simulink model "TosAeb"  (R2021a)
settings: Solver=FixedStepDiscrete  StartTime=0.0  StopTime=inf  FixedStep=0.01  RelTol=1e-3
undefined symbols (not defined in the file or in its folder): ACCModSts, AccSts1, AEB_ObjectMovingOrientation, AEB_ObjectMovingState, CN_BTCActive, CN_CurrentGearR, CN_CurrentGearR_DHT, CN_PTCActive, CN_VDCActive, CN_VSC_EspOff, CN_VSC_FCW_AEB_Error, CN_VSC_FCW_AEB_NoDispay, CN_VSC_FCW_AEB_PerfmDgdtn, CN_VSC_FCW_AEB_TempUnAvlbl, CN_VSC_VehStandstill, CNaccelAvoidMax, CNaccelAvoidMin, CNbrakePedalPress, CNcomfortStrLeftAccelMax, CNcomfortStrLeftAccelMin, CNcomfortStrRightAccelMax, CNcomfortStrRightAccelMin, CNDrvDoorOpen, CNDrvModeEco, CNDrvModeNorm, CNDrvModeOther, CNDrvModeSport, CNDrvSBRreminder, CNNull, CNobjInvalidID, CNPassDoorOpen, CNPassSBRreminder, CNPEBSAccelNegInf, CNPEBSAccelNull, CNPEBSTimeInf, CNPEBSTimeNull, CNSTOPMAX, CNSTOPNULL, CNsubjWidth, CNSwtOFF, CNSwtON, CNTTCMAX, CNTTCNULL, CNVehConfigAEBCycNone, CNVehConfigAEBNone, CNVehConfigAEBPedNone, CNVehConfigFCWNone, CNVehConfigJANone, CollisionSeverity, FALSE, FCWSnvty, JACycObjCalcInfoDefaultParams, JAPedObjCalcInfoDefaultParams, JAVehObjCalcInfoDefaultParams, latCycObjCalcInfoDefaultParams, latPedObjCalcInfoDefaultParams, longObjCalcInfoDefaultParams, ModSts, movingStatus, ObjectType ... (+1112)
libraries: simulink x3031, AEBSFunctionLib x50, Ta_Library x33, AEBSStateMachineLib x23, Mylibrary x12, simulink_extras x7, ObjSelect_lib x3, dspstat3 x2
(part 60 of 102)

== system TosAeb/FcwAebJa_Step_sys/ALG_AEBSystem/ALG_AEBController/AEB_FuncCondCalcApp/JaVehFunctionCondCalc/AEBJAVehAEBfullSuppAborCond/AEBJAVehAEBfullSuppCondCalc/AEBJAVehAEBfullSuppSubjCondCalcu/brakeReleventSuppCond/brakeReleventSuppCond_ESP ==
  ESP_MasterCylindBrakePress : Inport
  Action Port : ActionPort  ActionPortLabel=if(u1)
  Compare To Constant12 : simulink/Logic and Bit Operations/Compare To Constant  relop=>  const=P_AEB_Func_JaVehAEBfullMasterCylindBrkPressSupThres  OutDataTypeStr=boolean
  Constant : Constant  Value=P_AEB_Func_JaVehAEBfullBrkCondAborDelayTime
  Constant1 : Constant  Value=Ts
  StateDelayAbortion1 : AEBSFunctionLib/StateDelayAbortion  SystemSampleTime=-1  VariantActivationTime=update diagram  Latency=0  AutoFrameSizeCalculation=off
  brakeReleventSuppCond : Outport
  wires:
  Compare To Constant12/1 -> StateDelayAbortion1/1
  Constant1/1 -> StateDelayAbortion1/3
  Constant/1 -> StateDelayAbortion1/2
  StateDelayAbortion1/1 -> brakeReleventSuppCond/1  "BrkReleventSuppCondFulfilled"
  ESP_MasterCylindBrakePress/1 -> Compare To Constant12/1

== system TosAeb/FcwAebJa_Step_sys/ALG_AEBSystem/ALG_AEBController/AEB_FuncCondCalcApp/JaVehFunctionCondCalc/AEBJAVehAEBfullSuppAborCond/AEBJAVehAEBfullSuppCondCalc/AEBJAVehAEBfullSuppSubjCondCalcu/brakeReleventSuppCond/brakeReleventSuppCond_IBooster ==
  note: 0x0:Not applied
      | 0x1:Applied
  Action Port : ActionPort  ActionPortLabel=else
  Constant : Constant  Value=FALSE
  brakeReleventSuppCond : Outport
  wires:
  Constant/1 -> brakeReleventSuppCond/1  "brakeReleventSuppCond"

== system TosAeb/FcwAebJa_Step_sys/ALG_AEBSystem/ALG_AEBController/AEB_FuncCondCalcApp/JaVehFunctionCondCalc/AEBJAVehPrefillSuppAborCond/AEBJAVehPrefillSuppCondCalc/AEBJAVehPrefillSuppSubjCondCalcu/brakeReleventSuppCond/brakeReleventSuppCond_ESP ==
  BrakePedalStatus_G : Inport
  ESP_MasterCylindBrakePress : Inport  Port=2
  Action Port : ActionPort  ActionPortLabel=if(u1)
  Compare To Constant1 : simulink/Logic and Bit Operations/Compare To Constant  relop===  const=TRUE  OutDataTypeStr=boolean
  Compare To Constant12 : simulink/Logic and Bit Operations/Compare To Constant  relop=>  const=P_AEB_Func_JaVehPrefillMasterCylindBrkPressSupThres  OutDataTypeStr=boolean
  Constant : Constant  Value=P_AEB_Func_JaVehPrefillBrkCondAborDelayTime
  Constant1 : Constant  Value=Ts
  Logical Operator3 : Logic  Operator=OR  AllPortsSameDT=off  OutDataTypeStr=boolean
  StateDelayAbortion1 : AEBSFunctionLib/StateDelayAbortion  SystemSampleTime=-1  VariantActivationTime=update diagram  Latency=0  AutoFrameSizeCalculation=off
  brakeReleventSuppCond : Outport
  wires:
  Logical Operator3/1 -> brakeReleventSuppCond/1
  BrakePedalStatus_G/1 -> Compare To Constant1/1
  Constant1/1 -> StateDelayAbortion1/3
  Constant/1 -> StateDelayAbortion1/2
  StateDelayAbortion1/1 -> Logical Operator3/2
  ESP_MasterCylindBrakePress/1 -> Compare To Constant12/1
  Compare To Constant1/1 -> Logical Operator3/1
  Compare To Constant12/1 -> StateDelayAbortion1/1

== system TosAeb/FcwAebJa_Step_sys/ALG_AEBSystem/ALG_AEBController/AEB_FuncCondCalcApp/JaVehFunctionCondCalc/AEBJAVehPrefillSuppAborCond/AEBJAVehPrefillSuppCondCalc/AEBJAVehPrefillSuppSubjCondCalcu/brakeReleventSuppCond/brakeReleventSuppCond_IBooster ==
  note: 0x0:Not applied
      | 0x1:Applied
  IB_BrkPedalAppld : Inport
  Action Port : ActionPort  ActionPortLabel=else
  Compare To Constant3 : simulink/Logic and Bit Operations/Compare To Constant  relop===  const=TRUE  OutDataTypeStr=boolean
  brakeReleventSuppCond : Outport
  wires:
  IB_BrkPedalAppld/1 -> Compare To Constant3/1
  Compare To Constant3/1 -> brakeReleventSuppCond/1  "brakeReleventSuppCond"

== system TosAeb/FcwAebJa_Step_sys/ALG_AEBSystem/ALG_AEBController/AEB_FuncCondCalcApp/LongFunctionCondCalc/vehAEBfullSuppAborCondCalc/ vehAEBfullSuppCondCalc/vehAEBfullSituationRelActiveCondCalc/overlapThesCalc/If Action Subsystem ==
  dxFrontBumper : Inport
  ObjectFusion : ActionPort  ActionPortLabel=if(u1)
  OverlapThesLookupTable : Lookup_n-D  NumberOfTableDimensions=1  BreakpointsForDimension1=P_AEB_Func_vehAEBfullFusionOverlapActivationThresX  BeginIndexSearchUsingPreviousIndexResult=on  Table=P_AEB_Func_vehAEBfullFusionOverlapActivationThresY  ExtrapMethod=Clip  InternalRulePriority=Speed  RndMeth=Simplest  InputSameDT=on
  overlapThes : Outport
  wires:
  OverlapThesLookupTable/1 -> overlapThes/1
  dxFrontBumper/1 -> OverlapThesLookupTable/1

== system TosAeb/FcwAebJa_Step_sys/ALG_AEBSystem/ALG_AEBController/AEB_FuncCondCalcApp/LongFunctionCondCalc/vehAEBfullSuppAborCondCalc/ vehAEBfullSuppCondCalc/vehAEBfullSituationRelActiveCondCalc/overlapThesCalc/If Action Subsystem1 ==
  dxFrontBumper : Inport
  ObjectNoFusion : ActionPort  ActionPortLabel=else
  OverlapThesLookupTable : Lookup_n-D  NumberOfTableDimensions=1  BreakpointsForDimension1=P_AEB_Func_vehAEBfullNoFusionOverlapActivationThresX  BeginIndexSearchUsingPreviousIndexResult=on  Table=P_AEB_Func_vehAEBfullNoFusionOverlapActivationThresThresY  ExtrapMethod=Clip  InternalRulePriority=Speed  RndMeth=Simplest  InputSameDT=on
  overlapThes : Outport
  wires:
  dxFrontBumper/1 -> OverlapThesLookupTable/1
  OverlapThesLookupTable/1 -> overlapThes/1

== system TosAeb/FcwAebJa_Step_sys/ALG_AEBSystem/ALG_AEBController/AEB_FuncCondCalcApp/LongFunctionCondCalc/vehAEBfullSuppAborCondCalc/ vehAEBfullSuppCondCalc/vehAEBfullVehRelActiveCondCalc/DriverCondJudge/brakeReleventSuppCond ==
  VehConfigIbooster : Inport
  ESPmasterCylindBrakePress : Inport  Port=2
  BrakePedalStatus_G : Inport  Port=3
  subjLongAccel : Inport  Port=4
  subjVel : Inport  Port=5
  Compare To Constant : simulink/Logic and Bit Operations/Compare To Constant  relop===  const=FALSE  OutDataTypeStr=boolean
  If : If  IfExpression=u1
  Merge : Merge
  brakeReleventSuppCond_ESP : Subsystem  VariantControl=(default)
  brakeReleventSuppCond_IBooster : Subsystem  VariantControl=P_AEB_PowerTrainConfig == 1
  brakeReleventSuppCond : Outport
  wires:
  subjVel/1 -> brakeReleventSuppCond_ESP/4(subjVel)
  subjLongAccel/1 -> brakeReleventSuppCond_ESP/3(subjLongAccel)
  BrakePedalStatus_G/1 -> brakeReleventSuppCond_ESP/2(BrakePedalStatus_G)
  ESPmasterCylindBrakePress/1 -> brakeReleventSuppCond_ESP/1(ESPmasterCylindBrakePress)
  Merge/1 -> brakeReleventSuppCond/1
  brakeReleventSuppCond_IBooster/1(brakeReleventSuppCond) -> Merge/2
  brakeReleventSuppCond_ESP/1(brakeReleventSuppCond) -> Merge/1
  If/2 -> brakeReleventSuppCond_IBooster/Action
  If/1 -> brakeReleventSuppCond_ESP/Action
  Compare To Constant/1 -> If/1
  VehConfigIbooster/1 -> Compare To Constant/1

== system TosAeb/FcwAebJa_Step_sys/ALG_AEBSystem/ALG_AEBController/AEB_FuncCondCalcApp/LongFunctionCondCalc/vehAEBpartSuppAborCondCalc/vehAEBpartSuppCondCalc/vehAEBpartSituationRelActiveCondCalc/overlapThesCalc/If Action Subsystem ==
  dxFrontBumper : Inport
  ObjectFusion : ActionPort  ActionPortLabel=if(u1)
  OverlapThesLookupTable : Lookup_n-D  NumberOfTableDimensions=1  BreakpointsForDimension1=P_AEB_Func_vehAEBpartFusionOverlapActivationThresX  BeginIndexSearchUsingPreviousIndexResult=on  Table=P_AEB_Func_vehAEBpartFusionOverlapActivationThresY  ExtrapMethod=Clip  InternalRulePriority=Speed  RndMeth=Simplest  InputSameDT=on
  overlapThes : Outport
  wires:
  OverlapThesLookupTable/1 -> overlapThes/1
  dxFrontBumper/1 -> OverlapThesLookupTable/1

== system TosAeb/FcwAebJa_Step_sys/ALG_AEBSystem/ALG_AEBController/AEB_FuncCondCalcApp/LongFunctionCondCalc/vehAEBpartSuppAborCondCalc/vehAEBpartSuppCondCalc/vehAEBpartSituationRelActiveCondCalc/overlapThesCalc/If Action Subsystem1 ==
  dxFrontBumper : Inport
  ObjectNoFusion : ActionPort  ActionPortLabel=else
  OverlapThesLookupTable : Lookup_n-D  NumberOfTableDimensions=1  BreakpointsForDimension1=P_AEB_Func_vehAEBpartNoFusionOverlapActivationThresX  BeginIndexSearchUsingPreviousIndexResult=on  Table=P_AEB_Func_vehAEBpartNoFusionOverlapActivationThresY  ExtrapMethod=Clip  InternalRulePriority=Speed  RndMeth=Simplest  InputSameDT=on
  overlapThes : Outport
  wires:
  dxFrontBumper/1 -> OverlapThesLookupTable/1
  OverlapThesLookupTable/1 -> overlapThes/1

== system TosAeb/FcwAebJa_Step_sys/ALG_AEBSystem/ALG_AEBController/AEB_FuncCondCalcApp/LongFunctionCondCalc/vehAEBpartSuppAborCondCalc/vehAEBpartSuppCondCalc/vehAEBpartVehRelActiveCondCalc/DriverCondJudge/brakeReleventSuppCond ==
  VehConfigIbooster : Inport
  ESPmasterCylindBrakePress : Inport  Port=2
  BrakePedalStatus_G : Inport  Port=3
  IB_BrkPedalAppld : Inport  Port=4
  Compare To Constant : simulink/Logic and Bit Operations/Compare To Constant  relop===  const=FALSE  OutDataTypeStr=boolean
  If : If  IfExpression=u1
  Merge : Merge
  brakeReleventSuppCond_ESP : Subsystem  VariantControl=(default)
  brakeReleventSuppCond_IBooster : Subsystem  VariantControl=P_AEB_PowerTrainConfig == 1
  brakeReleventSuppCond : Outport
  wires:
  IB_BrkPedalAppld/1 -> brakeReleventSuppCond_IBooster/1(IB_BrkPedalAppld)
  ESPmasterCylindBrakePress/1 -> brakeReleventSuppCond_ESP/1(ESPmasterCylindBrakePress)
  BrakePedalStatus_G/1 -> brakeReleventSuppCond_ESP/2(BrakePedalStatus_G)
  Merge/1 -> brakeReleventSuppCond/1
  brakeReleventSuppCond_IBooster/1(brakeReleventSuppCond) -> Merge/2
  brakeReleventSuppCond_ESP/1(brakeReleventSuppCond) -> Merge/1
  If/2 -> brakeReleventSuppCond_IBooster/Action
  If/1 -> brakeReleventSuppCond_ESP/Action
  Compare To Constant/1 -> If/1
  VehConfigIbooster/1 -> Compare To Constant/1

== system TosAeb/FcwAebJa_Step_sys/ALG_AEBSystem/ALG_AEBController/AEB_FuncCondCalcApp/LongFunctionCondCalc/vehAcuteWarningSuppAborCondFulfilled/vehAcuteWarningSuppCondCalc/vehAcuteWarningVehRelActiveCondCalc/DriverCondJudge/brakeReleventSuppCond ==
  VehConfigIbooster : Inport
  ESPmasterCylindBrakePress : Inport  Port=2
  BrakePedalStatus_G : Inport  Port=3
  IB_BrkPedalAppld : Inport  Port=4
  Compare To Constant : simulink/Logic and Bit Operations/Compare To Constant  relop===  const=FALSE  OutDataTypeStr=boolean
  If : If  IfExpression=u1
  Merge : Merge
  brakeReleventSuppCond_ESP : Subsystem  VariantControl=(default)
  brakeReleventSuppCond_IBooster : Subsystem  VariantControl=P_AEB_PowerTrainConfig == 1
  brakeReleventSuppCond : Outport
  wires:
  IB_BrkPedalAppld/1 -> brakeReleventSuppCond_IBooster/1(IB_BrkPedalAppld)
  ESPmasterCylindBrakePress/1 -> brakeReleventSuppCond_ESP/1(ESPmasterCylindBrakePress)
  BrakePedalStatus_G/1 -> brakeReleventSuppCond_ESP/2(BrakePedalStatus_G)
  Merge/1 -> brakeReleventSuppCond/1
  brakeReleventSuppCond_IBooster/1(brakeReleventSuppCond) -> Merge/2
  brakeReleventSuppCond_ESP/1(brakeReleventSuppCond) -> Merge/1
  If/2 -> brakeReleventSuppCond_IBooster/Action
  If/1 -> brakeReleventSuppCond_ESP/Action
  Compare To Constant/1 -> If/1
  VehConfigIbooster/1 -> Compare To Constant/1

== system TosAeb/FcwAebJa_Step_sys/ALG_AEBSystem/ALG_AEBController/AEB_FuncCondCalcApp/LongFunctionCondCalc/vehEBASuppAborCondCalc/vehEBASuppCondCalc/vehEBASituationRelActiveCondCalc/brakeReleventSuppCond/brakeReleventSuppCond_ESP ==
  predictiveAccelAvoid : Inport
  ObjectMovingState : Inport  Port=2
  subjVel : Inport  Port=3
  ESPmasterCylindBrakePress : Inport  Port=4
  BrakePedalStatus_G : Inport  Port=5
  subjLongAccelFromSensor : Inport  Port=6
  subjLongAccel : Inport  Port=7
  Action Port : ActionPort  ActionPortLabel=if(u1)
  Constant2 : Constant  Value=P_AEB_Func_vehEBAMasterCylindBrkPressActivationThres
  Constant4 : Constant  Value=P_AEB_Func_vehEBAObjStandAccelAvoidThres
  Constant6 : Constant  Value=P_AEB_Func_vehEBAObjStandBrkAccelThresHigh
  Constant8 : Constant  Value=P_AEB_Func_vehEBAObjStandBrkAccelThresLow
  Constant9 : Constant  Value=P_AEB_Func_vehEBASubjLongAccelActivationThres
  brkTriggerCondCalc1 : Stateflow chart  TreatAsAtomicUnit=on
  brkTriggerCondFulfilled : Outport
  wires:
  ESPmasterCylindBrakePress/1 -> brkTriggerCondCalc1/4(ESPmasterCylindBrakePress)
  brkTriggerCondCalc1/1(brkTriggerCondFulfilled) -> brkTriggerCondFulfilled/1  "brkTriggerCondFulfilled"
  Constant8/1 -> brkTriggerCondCalc1/11(objStandBrkAccelThesLow)
  Constant2/1 -> brkTriggerCondCalc1/8(masterCylindBrkPressThes)
  ObjectMovingState/1 -> brkTriggerCondCalc1/2(objMovingState)
  Constant6/1 -> brkTriggerCondCalc1/10(objStandBrkAccelThesHigh)
  BrakePedalStatus_G/1 -> brkTriggerCondCalc1/5(brakePedalStatus)
  Constant4/1 -> brkTriggerCondCalc1/9(objStandAccelAvoidPredictThes)
  subjLongAccelFromSensor/1 -> brkTriggerCondCalc1/6(subjLongAccelFromSensor)
  Constant9/1 -> brkTriggerCondCalc1/12(subjLongAccelThes)
  subjLongAccel/1 -> brkTriggerCondCalc1/7(subjLongAccel)
  predictiveAccelAvoid/1 -> brkTriggerCondCalc1/1(predictiveAccelAvoid)
  subjVel/1 -> brkTriggerCondCalc1/3(subjVel)

== system TosAeb/FcwAebJa_Step_sys/ALG_AEBSystem/ALG_AEBController/AEB_FuncCondCalcApp/LongFunctionCondCalc/vehEBASuppAborCondCalc/vehEBASuppCondCalc/vehEBASituationRelActiveCondCalc/brakeReleventSuppCond/brakeReleventSuppCond_IBooster ==
  note: 0x0:Not applied
      | 0x1:Applied
  predictiveAccelAvoid : Inport
  ObjectMovingState : Inport  Port=2
  subjVel : Inport  Port=3
  subjLongAccelFromSensor : Inport  Port=4
  subjLongAccel : Inport  Port=5
  IB_BrkPedalAppld : Inport  Port=6
  Action Port : ActionPort  ActionPortLabel=else
  Constant4 : Constant  Value=P_AEB_Func_vehEBAObjStandAccelAvoidThres
  Constant6 : Constant  Value=P_AEB_Func_vehEBAObjStandBrkAccelThresHigh
  Constant8 : Constant  Value=P_AEB_Func_vehEBAObjStandBrkAccelThresLow
  Constant9 : Constant  Value=P_AEB_Func_vehEBASubjLongAccelActivationThres
  IB_BrkPedalAppldOpt : Subsystem
  brkAccelThesLookup : Subsystem  Commented=on
      settings: UndoDeleteFcn=Stateflow.SLINSF.SimulinkMan.restorePreDeleteFcn  PreDeleteFcn=Stateflow.SLINSF.SimulinkMan.removePreDeleteFcn
  brkTriggerCondCalc1 : Stateflow chart  TreatAsAtomicUnit=on
  brkTriggerCondFulfilled : Outport
  wires:
  subjLongAccel/1 -> brkTriggerCondCalc1/5(subjLongAccel)
  Constant4/1 -> brkTriggerCondCalc1/7(objStandAccelAvoidPredictThes)
  subjLongAccelFromSensor/1 -> brkTriggerCondCalc1/4(subjLongAccelFromSensor)
  brkTriggerCondCalc1/1(brkTriggerCondFulfilled) -> brkTriggerCondFulfilled/1  "brkTriggerCondFulfilled"
  Constant9/1 -> brkTriggerCondCalc1/10(subjLongAccelThes)
  Constant6/1 -> brkTriggerCondCalc1/8(objStandBrkAccelThesHigh)
  predictiveAccelAvoid/1 -> brkTriggerCondCalc1/1(predictiveAccelAvoid)
  Constant8/1 -> brkTriggerCondCalc1/9(objStandBrkAccelThesLow)
  ObjectMovingState/1 -> brkTriggerCondCalc1/2(objMovingState)
  subjVel/1 -> brkTriggerCondCalc1/3(subjVel)
  IB_BrkPedalAppld/1 -> IB_BrkPedalAppldOpt/1(IB_BrkPedalAppld)
  IB_BrkPedalAppldOpt/1(IB_BrkPedalAppldOpt) -> brkTriggerCondCalc1/6(IB_BrkPedalAppld)  "AEB_EbaIB_BrkPedalAppldOpt"

== system TosAeb/FcwAebJa_Step_sys/ALG_AEBSystem/ALG_AEBController/AEB_FuncCondCalcApp/LongFunctionCondCalc/vehEBASuppAborCondCalc/vehEBASuppCondCalc/vehEBASituationRelActiveCondCalc/overlapThesCalc/If Action Subsystem ==
  dxFrontBumper : Inport
  ObjectFusion : ActionPort  ActionPortLabel=if(u1)
  OverlapThesLookupTable : Lookup_n-D  NumberOfTableDimensions=1  BreakpointsForDimension1=P_AEB_Func_vehEBAFusionOverlapActivationThresX  BeginIndexSearchUsingPreviousIndexResult=on  Table=P_AEB_Func_vehEBAFusionOverlapActivationThresY  ExtrapMethod=Clip  InternalRulePriority=Speed  RndMeth=Simplest  InputSameDT=on
  overlapThes : Outport
  wires:
  OverlapThesLookupTable/1 -> overlapThes/1
  dxFrontBumper/1 -> OverlapThesLookupTable/1

== system TosAeb/FcwAebJa_Step_sys/ALG_AEBSystem/ALG_AEBController/AEB_FuncCondCalcApp/LongFunctionCondCalc/vehEBASuppAborCondCalc/vehEBASuppCondCalc/vehEBASituationRelActiveCondCalc/overlapThesCalc/If Action Subsystem1 ==
  dxFrontBumper : Inport
  ObjectNoFusion : ActionPort  ActionPortLabel=else
  OverlapThesLookupTable : Lookup_n-D  NumberOfTableDimensions=1  BreakpointsForDimension1=P_AEB_Func_vehEBANoFusionOverlapActivationThresX  BeginIndexSearchUsingPreviousIndexResult=on  Table=P_AEB_Func_vehEBANoFusionOverlapActivationThresY  ExtrapMethod=Clip  InternalRulePriority=Speed  RndMeth=Simplest  InputSameDT=on
  overlapThes : Outport
  wires:
  dxFrontBumper/1 -> OverlapThesLookupTable/1
  OverlapThesLookupTable/1 -> overlapThes/1

== system TosAeb/FcwAebJa_Step_sys/ALG_AEBSystem/ALG_AEBController/AEB_FuncCondCalcApp/LongFunctionCondCalc/vehLatentWarningSuppAborCondFulfilled/vehLatentWarningAborCondCalc/vehLatentWarningSituationRelAborCondCalc/timer/If Action Subsystem2 ==
  Action Port : ActionPort  InitializeStates=reset  ActionPortLabel=if(u1)
  Add : Sum
  Constant : Constant  Value=0.02
  Unit Delay : UnitDelay  InitialCondition=0.02  SampleTime=-1  HasFrameUpgradeWarning=on
  time : Outport
  wires:
  Constant/1 -> Add/2
  Add/1 -> Unit Delay/1
  Unit Delay/1 -> Add/1, time/1

== system TosAeb/FcwAebJa_Step_sys/ALG_AEBSystem/ALG_AEBController/AEB_FuncCondCalcApp/LongFunctionCondCalc/vehLatentWarningSuppAborCondFulfilled/vehLatentWarningAborCondCalc/vehLatentWarningSituationRelAborCondCalc/timer/If Action Subsystem3 ==
  Action Port : ActionPort  ActionPortLabel=else
  Constant : Constant  Value=0
  time : Outport
  wires:
  Constant/1 -> time/1

== system TosAeb/FcwAebJa_Step_sys/ALG_AEBSystem/ALG_AEBController/AEB_FuncCondCalcApp/LongFunctionCondCalc/vehPreWarningSuppAborCondFulfilled/vehPreWarningSuppCondCalc/vehPreWarningSituationRelActiveCondCalc/IsTtcCondFulfilledCalc/LowLvlBrkAccelThesCalcHMIEarly ==
  subjVel : Inport
  Action Port : ActionPort  ActionPortLabel=case [ HMISettingEarly ]:
  AEB_Func_VehPreWarning_TTC_Thres : Lookup_n-D  NumberOfTableDimensions=1  BreakpointsForDimension1=P_AEB_Func_vehPreWarning_TTC_ThresX4Eary  Table=P_AEB_Func_vehPreWarning_TTC_ThresY4Eary  ExtrapMethod=Clip  InternalRulePriority=Speed  RndMeth=Simplest  InputSameDT=on
  VehPreWarning_TTC_Thres : Outport
  wires:
  AEB_Func_VehPreWarning_TTC_Thres/1 -> VehPreWarning_TTC_Thres/1
  subjVel/1 -> AEB_Func_VehPreWarning_TTC_Thres/1

== system TosAeb/FcwAebJa_Step_sys/ALG_AEBSystem/ALG_AEBController/AEB_FuncCondCalcApp/LongFunctionCondCalc/vehPreWarningSuppAborCondFulfilled/vehPreWarningSuppCondCalc/vehPreWarningSituationRelActiveCondCalc/IsTtcCondFulfilledCalc/LowLvlBrkAccelThesCalcHMILate ==
  subjVel : Inport
  Action Port : ActionPort  ActionPortLabel=case [ HMISettingLate ]:
  AEB_Func_VehPreWarning_TTC_Thres : Lookup_n-D  NumberOfTableDimensions=1  BreakpointsForDimension1=P_AEB_Func_vehPreWarning_TTC_ThresX4Late  Table=P_AEB_Func_vehPreWarning_TTC_ThresY4Late  ExtrapMethod=Clip  InternalRulePriority=Speed  RndMeth=Simplest  InputSameDT=on
  VehPreWarning_TTC_Thres : Outport
  wires:
  AEB_Func_VehPreWarning_TTC_Thres/1 -> VehPreWarning_TTC_Thres/1
  subjVel/1 -> AEB_Func_VehPreWarning_TTC_Thres/1

== system TosAeb/FcwAebJa_Step_sys/ALG_AEBSystem/ALG_AEBController/AEB_FuncCondCalcApp/LongFunctionCondCalc/vehPreWarningSuppAborCondFulfilled/vehPreWarningSuppCondCalc/vehPreWarningSituationRelActiveCondCalc/IsTtcCondFulfilledCalc/LowLvlBrkAccelThesCalcHMIMid ==
  subjVel : Inport
  Action Port : ActionPort  ActionPortLabel=default:
  AEB_Func_VehPreWarning_TTC_Thres : Lookup_n-D  NumberOfTableDimensions=1  BreakpointsForDimension1=P_AEB_Func_vehPreWarning_TTC_ThresX4Mid  Table=P_AEB_Func_vehPreWarning_TTC_ThresY4Mid  ExtrapMethod=Clip  InternalRulePriority=Speed  RndMeth=Simplest  InputSameDT=on
  VehPreWarning_TTC_Thres : Outport
  wires:
  AEB_Func_VehPreWarning_TTC_Thres/1 -> VehPreWarning_TTC_Thres/1
  subjVel/1 -> AEB_Func_VehPreWarning_TTC_Thres/1

== system TosAeb/FcwAebJa_Step_sys/ALG_AEBSystem/ALG_AEBController/AEB_FuncCondCalcApp/LongFunctionCondCalc/vehPreWarningSuppAborCondFulfilled/vehPreWarningSuppCondCalc/vehPreWarningVehRelActiveCondCalc/DriverCondJudge/brakeReleventSuppCond ==
  VehConfigIbooster : Inport
  ESPmasterCylindBrakePress : Inport  Port=2
  BrakePedalStatus_G : Inport  Port=3
  IB_BrkPedalAppld : Inport  Port=4
  Compare To Constant : simulink/Logic and Bit Operations/Compare To Constant  relop===  const=FALSE  OutDataTypeStr=boolean
  If : If  IfExpression=u1
  Merge : Merge
  brakeReleventSuppCond_ESP : Subsystem  VariantControl=(default)
  brakeReleventSuppCond_IBooster : Subsystem  VariantControl=P_AEB_PowerTrainConfig == 1
  brakeReleventSuppCond : Outport
  wires:
  IB_BrkPedalAppld/1 -> brakeReleventSuppCond_IBooster/1(IB_BrkPedalAppld)
  ESPmasterCylindBrakePress/1 -> brakeReleventSuppCond_ESP/1(ESPmasterCylindBrakePress)
  BrakePedalStatus_G/1 -> brakeReleventSuppCond_ESP/2(BrakePedalStatus_G)
  Merge/1 -> brakeReleventSuppCond/1
  brakeReleventSuppCond_IBooster/1(brakeReleventSuppCond) -> Merge/2
  brakeReleventSuppCond_ESP/1(brakeReleventSuppCond) -> Merge/1
  If/2 -> brakeReleventSuppCond_IBooster/Action
  If/1 -> brakeReleventSuppCond_ESP/Action
  Compare To Constant/1 -> If/1
  VehConfigIbooster/1 -> Compare To Constant/1

== system TosAeb/FcwAebJa_Step_sys/ALG_AEBSystem/ALG_AEBController/AEB_FuncCondCalcApp/LongFunctionCondCalc/vehPrefillSuppAborCondCalc/vehPrefillSuppCondCalc/vehPrefillVehRelActiveCondCalc/DriverCondJudge/brakeReleventSuppCond ==
  VehConfigIbooster : Inport
  ESPmasterCylindBrakePress : Inport  Port=2
  BrakePedalStatus_G : Inport  Port=3
  IB_BrkPedalAppld : Inport  Port=4
  Compare To Constant : simulink/Logic and Bit Operations/Compare To Constant  relop===  const=FALSE  OutDataTypeStr=boolean
  If : If  IfExpression=u1
  Merge : Merge
  brakeReleventSuppCond_ESP : Subsystem  VariantControl=(default)
  brakeReleventSuppCond_IBooster : Subsystem  VariantControl=P_AEB_PowerTrainConfig == 1
  brakeReleventSuppCond : Outport
  wires:
  IB_BrkPedalAppld/1 -> brakeReleventSuppCond_IBooster/1(IB_BrkPedalAppld)
  ESPmasterCylindBrakePress/1 -> brakeReleventSuppCond_ESP/1(ESPmasterCylindBrakePress)
  BrakePedalStatus_G/1 -> brakeReleventSuppCond_ESP/2(BrakePedalStatus_G)
  Merge/1 -> brakeReleventSuppCond/1
  brakeReleventSuppCond_IBooster/1(brakeReleventSuppCond) -> Merge/2
  brakeReleventSuppCond_ESP/1(brakeReleventSuppCond) -> Merge/1
  If/2 -> brakeReleventSuppCond_IBooster/Action
  If/1 -> brakeReleventSuppCond_ESP/Action
  Compare To Constant/1 -> If/1
  VehConfigIbooster/1 -> Compare To Constant/1

== system TosAeb/FcwAebJa_Step_sys/ALG_AEBSystem/ALG_AEBController/AEB_FuncCondCalcApp/PedFunctionCondCalc/pedAEBfullSuppAborCond/pedAEBfullSuppCondCalc/pedAEBfullSituationRelSuppCondCalc/latDistCondCalc/LateralDistanceThresHMISettingEarly ==
  subjVel : Inport
  HMISettingEarlyOrMiddle : ActionPort  ActionPortLabel=case [ HMISettingEarly HMISettingMiddle ]:
  LateralDistanceThresLookupTable : Lookup_n-D  NumberOfTableDimensions=1  BreakpointsForDimension1=P_AEB_Func_pedAEBfullSubjVelLatBoundHmiEarlyMidX  BeginIndexSearchUsingPreviousIndexResult=on  Table=P_AEB_Func_pedAEBfullDyLatBoundHmiEarlyMidY  ExtrapMethod=Clip  InternalRulePriority=Speed  RndMeth=Simplest  InputSameDT=on
  latDistThres : Outport
  wires:
  subjVel/1 -> LateralDistanceThresLookupTable/1
  LateralDistanceThresLookupTable/1 -> latDistThres/1

== system TosAeb/FcwAebJa_Step_sys/ALG_AEBSystem/ALG_AEBController/AEB_FuncCondCalcApp/PedFunctionCondCalc/pedAEBfullSuppAborCond/pedAEBfullSuppCondCalc/pedAEBfullSituationRelSuppCondCalc/latDistCondCalc/LateralDistanceThresHMISettingLate ==
  vy : Inport
  HMISettingLate : ActionPort  ActionPortLabel=default:
  Abs : Abs
  LateralDistanceThresLookupTable : Lookup_n-D  NumberOfTableDimensions=1  BreakpointsForDimension1=P_AEB_Func_pedAEBfullObjVyAbsLatBoundHmiLateX  BeginIndexSearchUsingPreviousIndexResult=on  Table=P_AEB_Func_pedAEBfullDyLatBoundHmiLateY  ExtrapMethod=Clip  InternalRulePriority=Speed  RndMeth=Simplest  InputSameDT=on
  latDistThres : Outport
  wires:
  Abs/1 -> LateralDistanceThresLookupTable/1
  vy/1 -> Abs/1
  LateralDistanceThresLookupTable/1 -> latDistThres/1

== system TosAeb/FcwAebJa_Step_sys/ALG_AEBSystem/ALG_AEBController/AEB_FuncCondCalcApp/PedFunctionCondCalc/pedAEBfullSuppAborCond/pedAEBfullSuppCondCalc/pedAEBfullVehRelSuppCondCalc/brakeReleventSuppCond/brakeReleventSuppCond_ESP ==
  ESP_MasterCylindBrakePressValid : Inport
  ESP_MasterCylindBrakePress : Inport  Port=2
  Action Port : ActionPort  ActionPortLabel=if(u1)
  Compare To Constant11 : simulink/Logic and Bit Operations/Compare To Constant  relop===  const=TRUE  OutDataTypeStr=boolean
  Compare To Constant12 : simulink/Logic and Bit Operations/Compare To Constant  relop=>  const=P_AEB_Func_pedAEBfullMasterCylindBrkPressSupThres  OutDataTypeStr=boolean
  Logical Operator1 : Logic  AllPortsSameDT=off  OutDataTypeStr=boolean
  brakeReleventSuppCond : Outport
  wires:
  ESP_MasterCylindBrakePress/1 -> Compare To Constant12/1
  ESP_MasterCylindBrakePressValid/1 -> Compare To Constant11/1
  Compare To Constant11/1 -> Logical Operator1/1
  Logical Operator1/1 -> brakeReleventSuppCond/1
  Compare To Constant12/1 -> Logical Operator1/2

== system TosAeb/FcwAebJa_Step_sys/ALG_AEBSystem/ALG_AEBController/AEB_FuncCondCalcApp/PedFunctionCondCalc/pedAEBfullSuppAborCond/pedAEBfullSuppCondCalc/pedAEBfullVehRelSuppCondCalc/brakeReleventSuppCond/brakeReleventSuppCond_IBooster ==
  note: 0x0:Not applied
      | 0x1:Applied
  Action Port : ActionPort  ActionPortLabel=else
  Constant : Constant  Value=FALSE
  brakeReleventSuppCond : Outport
  wires:
  Constant/1 -> brakeReleventSuppCond/1  "brakeReleventSuppCond"

== system TosAeb/FcwAebJa_Step_sys/ALG_AEBSystem/ALG_AEBController/AEB_FuncCondCalcApp/PedFunctionCondCalc/pedPreWarningSuppAborCond/pedPreWarningSuppCondCalc/pedPreWarningVehRelActiveCondCalc/brakeReleventSuppCond/brakeReleventSuppCond_ESP ==
  ESP_MasterCylindBrakePressValid : Inport
  ESP_MasterCylindBrakePress : Inport  Port=2
  Action Port : ActionPort  ActionPortLabel=if(u1)
  Compare To Constant11 : simulink/Logic and Bit Operations/Compare To Constant  relop===  const=TRUE  OutDataTypeStr=boolean
  Compare To Constant12 : simulink/Logic and Bit Operations/Compare To Constant  relop=>  const=P_AEB_Func_pedPrewarningMasterCylindBrkPressSupThres  OutDataTypeStr=boolean
  Logical Operator1 : Logic  AllPortsSameDT=off  OutDataTypeStr=boolean
  brakeReleventSuppCond : Outport
  wires:
  Compare To Constant12/1 -> Logical Operator1/2
  ESP_MasterCylindBrakePressValid/1 -> Compare To Constant11/1
  Compare To Constant11/1 -> Logical Operator1/1
  Logical Operator1/1 -> brakeReleventSuppCond/1
  ESP_MasterCylindBrakePress/1 -> Compare To Constant12/1

== system TosAeb/FcwAebJa_Step_sys/ALG_AEBSystem/ALG_AEBController/AEB_FuncCondCalcApp/PedFunctionCondCalc/pedPreWarningSuppAborCond/pedPreWarningSuppCondCalc/pedPreWarningVehRelActiveCondCalc/brakeReleventSuppCond/brakeReleventSuppCond_IBooster ==
  note: 0x0:Not applied
      | 0x1:Applied
  IB_BrkPedalAppld : Inport
  Action Port : ActionPort  ActionPortLabel=else
  Compare To Constant3 : simulink/Logic and Bit Operations/Compare To Constant  relop===  const=TRUE  OutDataTypeStr=boolean
  brakeReleventSuppCond : Outport
  wires:
  IB_BrkPedalAppld/1 -> Compare To Constant3/1
  Compare To Constant3/1 -> brakeReleventSuppCond/1  "brakeReleventSuppCond"

== system TosAeb/FcwAebJa_Step_sys/ALG_AEBSystem/ALG_AEBController/AEB_FuncCondCalcApp/PedFunctionCondCalc/pedPrefillSuppAborCond/pedPrefillSuppCondCalc/pedPrefillVehRelActiveCondCalc/brakeReleventSuppCond/brakeReleventSuppCond_ESP ==
  ESP_MasterCylindBrakePressValid : Inport
  ESP_MasterCylindBrakePress : Inport  Port=2
  Action Port : ActionPort  ActionPortLabel=if(u1)
  Compare To Constant11 : simulink/Logic and Bit Operations/Compare To Constant  relop===  const=TRUE  OutDataTypeStr=boolean
  Compare To Constant12 : simulink/Logic and Bit Operations/Compare To Constant  relop=>  const=P_AEB_Func_pedPrefillMasterCylindBrkPressSupThres  OutDataTypeStr=boolean
  Logical Operator1 : Logic  AllPortsSameDT=off  OutDataTypeStr=boolean
  brakeReleventSuppCond : Outport
  wires:
  Compare To Constant11/1 -> Logical Operator1/1
  Logical Operator1/1 -> brakeReleventSuppCond/1
  ESP_MasterCylindBrakePress/1 -> Compare To Constant12/1
  ESP_MasterCylindBrakePressValid/1 -> Compare To Constant11/1
  Compare To Constant12/1 -> Logical Operator1/2

== system TosAeb/FcwAebJa_Step_sys/ALG_AEBSystem/ALG_AEBController/AEB_FuncCondCalcApp/PedFunctionCondCalc/pedPrefillSuppAborCond/pedPrefillSuppCondCalc/pedPrefillVehRelActiveCondCalc/brakeReleventSuppCond/brakeReleventSuppCond_IBooster ==
  note: 0x0:Not applied
      | 0x1:Applied
  IB_BrkPedalAppld : Inport
  Action Port : ActionPort  ActionPortLabel=else
  Compare To Constant3 : simulink/Logic and Bit Operations/Compare To Constant  relop===  const=TRUE  OutDataTypeStr=boolean
  brakeReleventSuppCond : Outport
  wires:
  IB_BrkPedalAppld/1 -> Compare To Constant3/1
  Compare To Constant3/1 -> brakeReleventSuppCond/1  "brakeReleventSuppCond"

== system TosAeb/FcwAebJa_Step_sys/ALG_AEBSystem/ALG_AEBController/AEB_FuncImpManagementApp/FuncManeuArbCalc/brkFuncManeuArbCalc/axTgtReqValueCalc1/AebfullTriggerInfoCalc/AEBTriggerRelCalc/AEBTriggerInfo ==
  FuncActvationTime : Inport
  AebActive : Inport  Port=2
  subjVel : Inport  Port=3
  vxPredicted : Inport  Port=4
  VSC_VehStandstill : Inport  Port=5
  AEBTriggerInfo : Stateflow chart  TreatAsAtomicUnit=on
  AebFullFuncStatus : Outport
  AebActiveFirstCycle : Outport  Port=2
  FuncActvationTimeAtStart : Outport  Port=3
  dxSecureAtStart : Outport  Port=4
  subjVelAtStart : Outport  Port=5
  vxPredictedAtStart : Outport  Port=6
  wires:
  AEBTriggerInfo/6(vxPredictedAtStart) -> vxPredictedAtStart/1
  FuncActvationTime/1 -> AEBTriggerInfo/2(FuncActvationTime)
  AEBTriggerInfo/4(dxSecureAtStart) -> dxSecureAtStart/1
  vxPredicted/1 -> AEBTriggerInfo/4(vxPredicted)
  AEBTriggerInfo/5(subjVelAtStart) -> subjVelAtStart/1
  AEBTriggerInfo/3(FuncActvationTimeAtStart) -> FuncActvationTimeAtStart/1
  AebActive/1 -> AEBTriggerInfo/1(AebActive)
  subjVel/1 -> AEBTriggerInfo/3(subjVel)
  VSC_VehStandstill/1 -> AEBTriggerInfo/5(VSC_VehStandstill)
  AEBTriggerInfo/2(AebActiveFirstCycle) -> AebActiveFirstCycle/1
  AEBTriggerInfo/1(AebFullFuncStatus) -> AebFullFuncStatus/1

== system TosAeb/FcwAebJa_Step_sys/ALG_AEBSystem/ALG_AEBController/AEB_FuncImpManagementApp/FuncManeuArbCalc/brkFuncManeuArbCalc/axTgtReqValueCalc1/AebfullTriggerInfoCalc/AEBTriggerRelCalc/FuncActvationTimeCalc ==
  AebActive : Inport
  Constant : Constant  Value=Ts
  Constant1 : Constant  Value=10
  Data Type Conversion : DataTypeConversion  OutDataTypeStr=single
  timer : AEBSFunctionLib/timer  SystemSampleTime=-1  VariantActivationTime=update diagram  Latency=0  AutoFrameSizeCalculation=off
  FuncActvationTime : Outport
  wires:
  Data Type Conversion/1 -> FuncActvationTime/1
  Constant1/1 -> timer/3
  Constant/1 -> timer/2
  timer/1 -> Data Type Conversion/1
  AebActive/1 -> timer/1

== system TosAeb/FcwAebJa_Step_sys/ALG_AEBSystem/ALG_AEBController/AEB_FuncImpManagementApp/FuncManeuArbCalc/brkFuncManeuArbCalc/axTgtReqValueCalc1/AebfullTriggerInfoCalc/BrakeInfo/If Action Subsystem ==
  Action Port : ActionPort  ActionPortLabel=if(u1)
  Constant5 : Constant  Value=P_AEB_FIM_VehAEBfullDecelGradientThresSO
  Constant6 : Constant  Value=P_AEB_FIM_VehAEBfullDecelMinThresSO
  JerkFuncDecMax : Outport
  accelMin : Outport  Port=2
  wires:
  Constant6/1 -> accelMin/1
  Constant5/1 -> JerkFuncDecMax/1

== system TosAeb/FcwAebJa_Step_sys/ALG_AEBSystem/ALG_AEBController/AEB_FuncImpManagementApp/FuncManeuArbCalc/brkFuncManeuArbCalc/axTgtReqValueCalc1/AebfullTriggerInfoCalc/BrakeInfo/If Action Subsystem1 ==
  subjVel : Inport  OutDataTypeStr=single
  Action Port : ActionPort  ActionPortLabel=else
  Constant4 : Constant  Value=P_AEB_FIM_VehAEBfullDecelGradientThresMO
  MaxDecelLookupTable : Lookup_n-D  NumberOfTableDimensions=1  BreakpointsForDimension1=P_AEB_FIM_VehAEBfullDecelMinThresMOX  BeginIndexSearchUsingPreviousIndexResult=on  Table=P_AEB_FIM_VehAEBfullDecelMinThresMOY  ExtrapMethod=Clip  InternalRulePriority=Speed  OutDataTypeStr=single  RndMeth=Simplest  InputSameDT=on
  JerkFuncDecMax : Outport
  accelMin : Outport  Port=2
  wires:
  subjVel/1 -> MaxDecelLookupTable/1
  Constant4/1 -> JerkFuncDecMax/1
  MaxDecelLookupTable/1 -> accelMin/1

== system TosAeb/FcwAebJa_Step_sys/ALG_AEBSystem/ALG_AEBController/AEB_FuncImpManagementApp/FuncManeuArbCalc/brkFuncManeuArbCalc/axTgtReqValueCalc1/AebfullTriggerInfoCalc/Subsystem/If Action Subsystem ==
  Action Port : ActionPort  ActionPortLabel=if(u1)
  Bus Creator : BusCreator  Inputs=3  OutDataTypeStr=Bus: BusFIM_ObjPredicted  NonVirtualBus=on
      settings: InheritFromInputs=off
  Constant : Constant  Value=CNNull
  Constant1 : Constant  Value=CNNull
  Constant2 : Constant  Value=CNNull
  ObjectPredicted : Outport
  wires:
  Bus Creator/1 -> ObjectPredicted/1
  Constant2/1 -> Bus Creator/3  "vxPredicted"
  Constant/1 -> Bus Creator/1  "dxFrontBumperPredicted"
  Constant1/1 -> Bus Creator/2  "vxAbsObjPredicted"

== system TosAeb/FcwAebJa_Step_sys/ALG_AEBSystem/ALG_AEBController/AEB_FuncImpManagementApp/FuncManeuArbCalc/brkFuncManeuArbCalc/axTgtReqValueCalc1/AebfullTriggerInfoCalc/Subsystem/ObjectPredict ==
  subjVel : Inport
  dxFrontBumper : Inport  Port=2
  vx : Inport  Port=3
  vxAbsObj : Inport  Port=4
  ax : Inport  Port=5
  Action Port : ActionPort  ActionPortLabel=else
  Constant : Constant  Value=P_AEB_ObjectPredictConfig4Control
  If : If  IfExpression=u1
  If Action Subsystem : Subsystem  TreatAsAtomicUnit=on
  If Action Subsystem1 : Subsystem  TreatAsAtomicUnit=on
  Merge : Merge
  ObjectPredicted : Outport  OutDataTypeStr=Bus: BusFIM_ObjPredicted
  wires:
  If/1 -> If Action Subsystem/Action
  Constant/1 -> If/1
  If Action Subsystem/1(ObjectPredicted) -> Merge/1
  Merge/1 -> ObjectPredicted/1
  If/2 -> If Action Subsystem1/Action
  If Action Subsystem1/1(ObjectPredicted) -> Merge/2
  subjVel/1 -> If Action Subsystem/1(subjVel)  "subjVel"
  dxFrontBumper/1 -> [dxFrontBumper], If Action Subsystem/2(dxFrontBumper)  "dxFrontBumper"
  vx/1 -> [vx], If Action Subsystem/3(vx)  "vx"
  ax/1 -> If Action Subsystem/4(ax)  "ax"
  vxAbsObj/1 -> If Action Subsystem1/1(vxAbsObj)
  [dxFrontBumper] -> If Action Subsystem1/2(dxFrontBumper)
  [vx] -> If Action Subsystem1/3(vx)

== system TosAeb/FcwAebJa_Step_sys/ALG_AEBSystem/ALG_AEBController/AEB_FuncImpManagementApp/FuncManeuArbCalc/brkFuncManeuArbCalc/axTgtReqValueCalc1/AebfullTriggerInfoCalc/axAbsObjFiltCalc/LowPassFilter1 ==
  x : Inport
  Add : Sum
  Add1 : Sum  Inputs=+-
  Constant : Constant  Value=FiltCoef
  Constant1 : Constant  OutDataTypeStr=single
  Constant2 : Constant  Value=FiltCoef
  Delay : Delay  DelayLength=1  InitialConditionSource=Input port
      settings: InputPortMap=u0,p4
  Product : Product
  Product1 : Product
  y : Outport
  wires:
  Constant2/1 -> Add1/2
  Delay/1 -> Product1/2
  Add1/1 -> Product1/1
  Add/1 -> y/1, Delay/1
  Constant1/1 -> Add1/1
  Product/1 -> Add/1
  Constant/1 -> Product/2
  x/1 -> Delay/2, Product/1
  Product1/1 -> Add/2

== system TosAeb/FcwAebJa_Step_sys/ALG_AEBSystem/ALG_AEBController/AEB_FuncImpManagementApp/FuncManeuArbCalc/brkFuncManeuArbCalc/axTgtReqValueCalc1/Fctb_IsNeedBrakeCalc/IsNeedBrakeFlow/Distance_Cal2/If Action Subsystem ==
  Action Port : ActionPort  ActionPortLabel=if(u1)
  Constant : Constant  Value=10  OutDataTypeStr=single
  Distance2 : Outport
  wires:
  Constant/1 -> Distance2/1

== system TosAeb/FcwAebJa_Step_sys/ALG_AEBSystem/ALG_AEBController/AEB_FuncImpManagementApp/FuncManeuArbCalc/brkFuncManeuArbCalc/axTgtReqValueCalc1/Fctb_IsNeedBrakeCalc/IsNeedBrakeFlow/Distance_Cal2/Subsystem1 ==
  Fctb_AxReq : Inport  OutDataTypeStr=single
  subjVel : Inport  Port=2  OutDataTypeStr=single
  Action Port : ActionPort  ActionPortLabel=else
  Abs : Abs
  Constant1 : Constant  Value=10  OutDataTypeStr=single
  Constant2 : Constant  Value=CNNull
  Divide : Product  Inputs=*/
  Max : MinMax  Function=max  Inputs=2
  Min : MinMax  Inputs=2
  Product : Product
  Distance2 : Outport
  wires:
  Product/1 -> Divide/1
  subjVel/1 -> Product/2, Product/1
  Fctb_AxReq/1 -> Abs/1
  Constant2/1 -> Max/2
  Constant1/1 -> Min/2
  Divide/1 -> Min/1
  Min/1 -> Max/1
  Max/1 -> Distance2/1
  Abs/1 -> Divide/2

== system TosAeb/FcwAebJa_Step_sys/ALG_AEBSystem/ALG_AEBController/AEB_FuncImpManagementApp/FuncManeuArbCalc/brkFuncManeuArbCalc/axTgtReqValueCalc1/axTgtReqValueCalc4AebControl/JerkFuncDecCalc/GradientFuncDecK1Opt ==
  aDesired : Inport
  axTgtReqValueK1 : Inport  Port=2
  FuncActvationTime : Inport  Port=3
  FuncActvationTimeAtStart : Inport  Port=4
  vxAtStart : Inport  Port=5
  1-D Lookup Table : Lookup_n-D  NumberOfTableDimensions=1  BreakpointsForDimension1=P_AEB_FIM_JerkTimeHoldX_vRelStart  Table=P_AEB_FIM_JerkHoldTimeY  ExtrapMethod=Clip  InternalRulePriority=Speed  RndMeth=Simplest  InputSameDT=on
  Add : Sum
  Compare To Constant : simulink/Logic and Bit Operations/Compare To Constant  relop=>  const=P_AEB_FIM_aErroraDesiredaTgtThres4JerkReset  OutDataTypeStr=boolean
  Logical Operator : Logic  AllPortsSameDT=off  OutDataTypeStr=boolean
  Relational Operator : RelationalOperator  OutDataTypeStr=boolean  RndMeth=Simplest
  Subtract : Sum  Inputs=+-
  JerkFuncDecReset : Outport
  JerkDecHoldTime : Outport  Port=2
  wires:
  Relational Operator/1 -> Logical Operator/2, JerkDecHoldTime/1  "AEB_FIM_JerkDecHoldTime"
  Compare To Constant/1 -> Logical Operator/1
  FuncActvationTime/1 -> Relational Operator/1
  Add/1 -> Relational Operator/2
  1-D Lookup Table/1 -> Add/2
  vxAtStart/1 -> 1-D Lookup Table/1
  FuncActvationTimeAtStart/1 -> Add/1
  Subtract/1 -> Compare To Constant/1
  axTgtReqValueK1/1 -> Subtract/2
  aDesired/1 -> Subtract/1
  Logical Operator/1 -> JerkFuncDecReset/1  "AEB_JerkFuncDecReset"

== system TosAeb/FcwAebJa_Step_sys/ALG_AEBSystem/ALG_AEBController/AEB_FuncImpManagementApp/FuncManeuArbCalc/brkFuncManeuArbCalc/axTgtReqValueCalc1/axTgtReqValueCalc4AebControl/JerkFuncDecCalc/JerkFuncDecCalc ==
  JerkFuncDecMax : Inport
  aDesired : Inport  Port=2
  accelMin : Inport  Port=3
  axTgtReqValueK1 : Inport  Port=4
  AebActiveFirstCycle : Inport  Port=5
  JerkFuncDecReset : Inport  Port=6
  JerkDecHoldTime : Inport  Port=7
  JerkFuncDecCalc : Stateflow chart  TreatAsAtomicUnit=on
  JerkFuncDec : Outport
  wires:
  JerkFuncDecCalc/1(JerkFuncDec) -> JerkFuncDec/1
  JerkDecHoldTime/1 -> JerkFuncDecCalc/6(JerkDecHoldTime)
  JerkFuncDecReset/1 -> JerkFuncDecCalc/1(JerkFuncDecReset)
  accelMin/1 -> JerkFuncDecCalc/4(accelMin)
  axTgtReqValueK1/1 -> JerkFuncDecCalc/5(axTgtReqValueK1)
  aDesired/1 -> JerkFuncDecCalc/3(aDesired)
  JerkFuncDecMax/1 -> JerkFuncDecCalc/2(JerkFuncDecMax)
  AebActiveFirstCycle/1 -> JerkFuncDecCalc/7(AebActiveFirstCycle)

== system TosAeb/FcwAebJa_Step_sys/ALG_AEBSystem/ALG_AEBController/AEB_FuncImpManagementApp/FuncManeuArbCalc/brkFuncManeuArbCalc/axTgtReqValueCalc1/axTgtReqValueCalc4AebControl/JerkFuncIncCalc/IncPhaseCalc ==
  aErrorDesiredTgtValue : Inport
  dxFrontBumperPredicted : Inport  Port=2
  AebActiveFirstCycle : Inport  Port=3
  Chart : Stateflow chart  TreatAsAtomicUnit=on
  Constant : Constant  Value=P_AEB_FIM_aErrorThres4BrakeStage1
  Constant1 : Constant  Value=P_AEB_FIM_aErrorThres4BrakeStage2
  Constant2 : Constant  Value=P_AEB_FIM_aErrorThres4BrakeStage3
  Constant3 : Constant  Value=P_AEB_FIM_aErrorThres4BrakeStage4
  Constant4 : Constant  Value=P_AEB_FIM_aErrorThres4BrakeStage5
  Constant5 : Constant  Value=Ts
  Constant6 : Constant  Value=P_AEB_FIM_aErrorThres4BrakeStage6
  Constant7 : Constant  Value=P_AEB_FIM_distMinThres4LowControlling
  Constant8 : Constant  Value=P_AEB_FIM_TimerNumber4JerkInc
  ReachedIncStage : Outport
  currentIncStage : Outport  Port=2
  time4IncControlling : Outport  Port=3
  wires:
  Constant7/1 -> Chart/11(P_AEB_FIM_distMinThres4LowControlling)  "P_AEB_FIM_distMinThres4LowControlling"
  Constant6/1 -> Chart/10(P_AEB_FIM_aDeltaThres4BrakeStage6)  "P_AEB_FIM_aErrorThres4BrakeStage6"
  Constant4/1 -> Chart/9(P_AEB_FIM_aErrorThres4BrakeStage5)  "P_AEB_FIM_aErrorThres4BrakeStage5"
  Constant3/1 -> Chart/8(P_AEB_FIM_aErrorThres4BrakeStage4)  "P_AEB_FIM_aErrorThres4BrakeStage4"
  Constant/1 -> Chart/5(P_AEB_FIM_aErrorThres4BrakeStage1)  "P_AEB_FIM_aErrorThres4BrakeStage1"
  Constant1/1 -> Chart/6(P_AEB_FIM_aErrorThres4BrakeStage2)  "P_AEB_FIM_aErrorThres4BrakeStage2"
  Constant2/1 -> Chart/7(P_AEB_FIM_aErrorThres4BrakeStage3)  "P_AEB_FIM_aErrorThres4BrakeStage3"
  aErrorDesiredTgtValue/1 -> Chart/1(aErrorDesiredTgtValue)
  dxFrontBumperPredicted/1 -> Chart/2(dxFrontBumperPredicted)
  Chart/1(ReachedIncStage) -> ReachedIncStage/1
  Chart/2(currentIncStage) -> currentIncStage/1
  Chart/3(time4IncControlling) -> time4IncControlling/1
  Constant5/1 -> Chart/4(Ts)
  Constant8/1 -> Chart/12(P_AEB_FIM_TimerNumber4JerkInc)  "P_AEB_FIM_TimerNumber4GradientInc"
  AebActiveFirstCycle/1 -> Chart/3(AebActiveFirstCycle)

== system TosAeb/FcwAebJa_Step_sys/ALG_AEBSystem/ALG_AEBController/AEB_FuncImpManagementApp/FuncManeuArbCalc/brkFuncManeuArbCalc/axTgtReqValueCalc1/axTgtReqValueCalc4AebControl/JerkFuncIncCalc/JerkFuncIncCalc ==
  time4IncControlling : Inport
  longBrakingFlg : Inport  Port=2
  Compare To Constant : simulink/Logic and Bit Operations/Compare To Constant  relop===  const=false  OutDataTypeStr=boolean
  If : If  IfExpression=u1
  JerkFuncInc4LongBraking : Subsystem  TreatAsAtomicUnit=on
  JerkFuncIncAbs : Subsystem
  JerkFuncIncCalc : Subsystem  TreatAsAtomicUnit=on
  Merge : Merge
  JerkFuncInc : Outport
  wires:
  Compare To Constant/1 -> If/1
  If/1 -> JerkFuncInc4LongBraking/Action
  longBrakingFlg/1 -> Compare To Constant/1
  time4IncControlling/1 -> JerkFuncIncCalc/1(time4IncConstant), JerkFuncInc4LongBraking/1(time4IncConstant)
  If/2 -> JerkFuncIncCalc/Action
  JerkFuncInc4LongBraking/1(JerkFuncInc) -> Merge/1
  JerkFuncIncCalc/1(JerkFuncInc) -> Merge/2
  Merge/1 -> JerkFuncIncAbs/1(JerkFuncInc)
  JerkFuncIncAbs/1(JerkFuncIncAbs) -> JerkFuncInc/1

== system TosAeb/FcwAebJa_Step_sys/ALG_AEBSystem/ALG_AEBController/AEB_FuncImpManagementApp/FuncManeuArbCalc/brkFuncManeuArbCalc/axTgtReqValueCalc1/axTgtReqValueCalc4AebControl/JerkFuncIncCalc/aErrorDesiredTgtValueCalc ==
  axTgtReqValueK1 : Inport
  aDesired : Inport  Port=2
  Subtract : Sum  Inputs=+-
  aErrorDesiredTgtValue : Outport
  wires:
  axTgtReqValueK1/1 -> Subtract/1
  aDesired/1 -> Subtract/2
  Subtract/1 -> aErrorDesiredTgtValue/1

== system TosAeb/FcwAebJa_Step_sys/ALG_AEBSystem/ALG_AEBController/AEB_FuncImpManagementApp/FuncManeuArbCalc/brkFuncManeuArbCalc/axTgtReqValueCalc1/axTgtReqValueCalc4AebControl/JerkFuncIncCalc/longBrakingFlg ==
  axAbsObj : Inport
  vxPredictedAtStart : Inport  Port=2
  Compare To Constant : simulink/Logic and Bit Operations/Compare To Constant  relop=<  const=P_AEB_FIM_axAbsObjThres4LongBrakingOn  OutDataTypeStr=boolean
  Compare To Constant1 : simulink/Logic and Bit Operations/Compare To Constant  relop=>  const=P_AEB_FIM_axAbsObjThres4LongBrakingOff  OutDataTypeStr=boolean
  Compare To Constant2 : simulink/Logic and Bit Operations/Compare To Constant  relop=<  const=P_AEB_FIM_VxThres4LongBraking  OutDataTypeStr=boolean
  Constant : Constant  Value=true
  Constant1 : Constant  Value=false
  Logical Operator : Logic  Operator=OR  AllPortsSameDT=off  OutDataTypeStr=boolean
  Switch : Switch  Criteria=u2 ~= 0
  Switch1 : Switch  Criteria=u2 ~= 0
  Unit Delay : UnitDelay  SampleTime=-1  HasFrameUpgradeWarning=on
  longBrakingFlg : Outport
  wires:
  axAbsObj/1 -> Compare To Constant1/1, Compare To Constant/1
  Compare To Constant/1 -> Switch/2
  Compare To Constant1/1 -> Switch1/2
  Switch1/1 -> Switch/3
  Constant/1 -> Switch/1
  Constant1/1 -> Switch1/1
  Switch/1 -> Unit Delay/1, Logical Operator/1
  Unit Delay/1 -> Switch1/3
  vxPredictedAtStart/1 -> Compare To Constant2/1
  Compare To Constant2/1 -> Logical Operator/2
  Logical Operator/1 -> longBrakingFlg/1

== system TosAeb/FcwAebJa_Step_sys/ALG_AEBSystem/ALG_AEBController/AEB_FuncImpManagementApp/FuncManeuArbCalc/brkFuncManeuArbCalc/axTgtReqValueCalc1/axTgtReqValueCalc4AebControl/aDesiredCalc/If Action Subsystem ==
  accelMin : Inport
  Action Port : ActionPort  ActionPortLabel=if(u1)
  Add : Sum
  Constant : Constant  Value=-0.5  OutDataTypeStr=single
  accelAvoid : Outport
  wires:
  Add/1 -> accelAvoid/1
  Constant/1 -> Add/2
  accelMin/1 -> Add/1

== system TosAeb/FcwAebJa_Step_sys/ALG_AEBSystem/ALG_AEBController/AEB_FuncImpManagementApp/FuncManeuArbCalc/brkFuncManeuArbCalc/axTgtReqValueCalc1/axTgtReqValueCalc4AebControl/aDesiredCalc/If Action Subsystem1 ==
  vx : Inport
  axAbsObj : Inport  Port=2
  vxAbsObj : Inport  Port=3
  dxFrontBumper : Inport  Port=4
  subjVel : Inport  Port=5
  dxSecure : Inport  Port=6
  Action Port : ActionPort  ActionPortLabel=else
  accelAvoidLowLvlBrkCalc : Subsystem  TreatAsAtomicUnit=on
  dxEgoBrakeCalc : Subsystem
  tObjStopCalc : Subsystem  TreatAsAtomicUnit=on
  accelAvoid : Outport
  wires:
  accelAvoidLowLvlBrkCalc/1(accelAvoidLowLvlBrk) -> accelAvoid/1
  dxEgoBrakeCalc/1(distEgoBrake) -> accelAvoidLowLvlBrkCalc/6(dxEgoBrake)
  dxFrontBumper/1 -> dxEgoBrakeCalc/1(dxFrontBumper)
  vx/1 -> accelAvoidLowLvlBrkCalc/1(vx)
  vxAbsObj/1 -> tObjStopCalc/2(velAbs), accelAvoidLowLvlBrkCalc/4(vxAbsObj)
  tObjStopCalc/1(tStop) -> accelAvoidLowLvlBrkCalc/3(tObjStop)  "tObjStop"
  subjVel/1 -> accelAvoidLowLvlBrkCalc/5(subjVel)
  dxSecure/1 -> dxEgoBrakeCalc/2(dxSecure)
  axAbsObj/1 -> accelAvoidLowLvlBrkCalc/2(axAbsObj), tObjStopCalc/1(accelAbs)

== system TosAeb/FcwAebJa_Step_sys/ALG_AEBSystem/ALG_AEBController/AEB_FuncImpManagementApp/FuncManeuArbCalc/brkFuncManeuArbCalc/axTgtReqValueCalc1/axTgtReqValueCalc4AebControl/aDesiredCalc/accelAvoidFilt ==
  AebActiveFirstCycle : Inport
  accelAvoid : Inport  Port=2
  Add : Sum
  Compare To Constant : simulink/Logic and Bit Operations/Compare To Constant  relop===  const=TRUE  OutDataTypeStr=boolean
  Gain : Gain  Gain=0.5
  Switch : Switch  Criteria=u2 ~= 0
  Unit Delay : UnitDelay  SampleTime=-1  HasFrameUpgradeWarning=on
  aDesired : Outport
  wires:
  Add/1 -> Gain/1
  accelAvoid/1 -> Add/1, Switch/1
  Unit Delay/1 -> Add/2
  AebActiveFirstCycle/1 -> Compare To Constant/1
  Switch/1 -> aDesired/1, Unit Delay/1
  Gain/1 -> Switch/3
  Compare To Constant/1 -> Switch/2

== system TosAeb/FcwAebJa_Step_sys/ALG_AEBSystem/ALG_AEBController/AEB_FuncImpManagementApp/FuncManeuArbCalc/brkFuncManeuArbCalc/axTgtReqValueCalc1/axTgtReqValueCalc4AebControl/aDesiredCalc/underDxSecure ==
  dxFrontBumperPredicted : Inport
  dxSecure : Inport  Port=2
  vxPredicted : Inport  Port=3
  subjVel : Inport  Port=4
  Abs : Abs
  Add : Sum
  Compare To Constant : simulink/Logic and Bit Operations/Compare To Constant  relop=<=  const=P_AEB_FIM_VxThres4underDxSecure  OutDataTypeStr=boolean
  Compare To Constant1 : simulink/Logic and Bit Operations/Compare To Constant  relop=<=  const=P_AEB_FIM_SubjVelThres4underDxSecure  OutDataTypeStr=boolean
  Constant : Constant  Value=P_AEB_FIM_dxOffset4underDxSecure
  Constant1 : Constant  Value=FALSE
  Logical Operator1 : Logic  Operator=OR  AllPortsSameDT=off  OutDataTypeStr=boolean
  Relational Operator : RelationalOperator  Operator=<=  OutDataTypeStr=boolean  RndMeth=Simplest
  Switch : Switch  Criteria=u2 ~= 0
  underDxSecure : Outport
  wires:
  Switch/1 -> underDxSecure/1  "AEB_FIM_underDxSecure"
  dxSecure/1 -> Add/1
  dxFrontBumperPredicted/1 -> Relational Operator/1
  Constant/1 -> Add/2
  Add/1 -> Relational Operator/2
  vxPredicted/1 -> Abs/1
  Compare To Constant/1 -> Logical Operator1/1
  subjVel/1 -> Compare To Constant1/1
  Compare To Constant1/1 -> Logical Operator1/2
  Logical Operator1/1 -> Switch/2
  Constant1/1 -> Switch/1
  Relational Operator/1 -> Switch/3
  Abs/1 -> Compare To Constant/1

== system TosAeb/FcwAebJa_Step_sys/ALG_AEBSystem/ALG_AEBController/AEB_FuncImpManagementApp/FuncManeuArbCalc/brkFuncManeuArbCalc/axTgtReqValueCalc1/axTgtReqValueCalc4AebControl/axTgtReqValueCalc/axTgtReqValueFinal ==
  axTgtReqValueK1 : Inport
  JerkFuncInc : Inport  Port=2
  JerkFuncDec : Inport  Port=3
  aDesired : Inport  Port=4
  accelMax : Inport  Port=5
  accelMin : Inport  Port=6
  Action Port : ActionPort  ActionPortLabel=else
  Constant : Constant  Value=Ts
  axTgtReqValueCalc : Subsystem  TreatAsAtomicUnit=on
  axTgtReqValue : Outport
  wires:
  JerkFuncInc/1 -> axTgtReqValueCalc/1(JerkFuncInc)
  axTgtReqValueK1/1 -> axTgtReqValueCalc/5(axTgtReqValueK1)
  JerkFuncDec/1 -> axTgtReqValueCalc/2(JerkFuncDec)
  axTgtReqValueCalc/1(axTgtReqValue) -> axTgtReqValue/1
  accelMin/1 -> axTgtReqValueCalc/7(accelMin)
  aDesired/1 -> axTgtReqValueCalc/4(aDesired)
  Constant/1 -> axTgtReqValueCalc/3(Ts)
  accelMax/1 -> axTgtReqValueCalc/6(accelMax)
